simulink library "Garbage"  (R2023a) (continued)
== system Garbage/NOT_USED_Decoder_8_to_256 == (continued)
  xyz1/5 -> Lo`gical Operator6/1, Logical Operator127/5, Logical Operator1/5, Logical Operator2/5, Logical Operator3/5, Logical Operator4/5, Logical Operator5/5, Logical Operator6/5, Logical Operator7/5, Logical Operator256/5, Logical Operator20/5, Logical Operator16/5, Logical Operator24/5, Logical Operator25/5, Logical Operator26/5, Logical Operator27/5, Logical Operator28/5, Logical Operator22/5, Logical Operator23/5, Logical Operator36/5, Logical Operator32/5, Logical Operator40/5, Logical Operator41/5, Logical Operator42/5, Logical Operator43/5, Logical Operator37/5, Logical Operator38/5, Logical Operator39/5, Logical Operator52/5, Logical Operator48/5, Logical Operator56/5, Logical Operator57/5, Logical Operator58/5, Logical Operator53/5, Logical Operator54/5, Logical Operator55/5, Logical Operator68/5, Logical Operator64/5, Logical Operator253/5, Logical Operator76/5, Logical Operator89/5, Logical Operator67/5, Logical Operator69/5, Logical Operator70/5, Logical Operator71/5, Logical Operator77/5, Logical Operator72/5, Logical Operator81/5, Logical Operator84/5, Logical Operator74/5, Logical Operator75/5, Logical Operator78/5, Logical Operator79/5, Logical Operator80/5, Logical Operator96/5, Logical Operator92/5, Logical Operator101/5, Logical Operator93/5, Logical Operator94/5, Logical Operator95/5, Logical Operator97/5, Logical Operator98/5, Logical Operator99/5, Logical Operator115/5, Logical Operator109/5, Logical Operator113/5, Logical Operator249/5, Logical Operator114/5, Logical Operator116/5, Logical Operator117/5, Logical Operator118/5, Logical Operator136/5, Logical Operator195/5, Logical Operator133/5, Logical Operator134/5, Logical Operator135/5, Logical Operator137/5, Logical Operator138/5, Logical Operator139/5, Logical Operator145/5, Logical Operator156/5, Logical Operator157/5, Logical Operator141/5, Logical Operator142/5, Logical Operator143/5, Logical Operator146/5, Logical Operator147/5, Logical Operator148/5, Logical Operator172/5, Logical Operator173/5, Logical Operator174/5, Logical Operator159/5, Logical Operator160/5, Logical Operator161/5, Logical Operator163/5, Logical Operator164/5, Logical Operator190/5, Logical Operator245/5, Logical Operator191/5, Logical Operator192/5, Logical Operator176/5, Logical Operator178/5, Logical Operator179/5, Logical Operator181/5, Logical Operator252/5, Logical Operator257/5, Logical Operator244/5, Logical Operator258/5, Logical Operator259/5, Logical Operator197/5, Logical Operator198/5, Logical Operator199/5, Logical Operator216/5, Logical Operator217/5, Logical Operator218/5, Logical Operator220/5, Logical Operator221/5, Logical Operator205/5, Logical Operator243/5, Logical Operator206/5, Logical Operator233/5, Logical Operator234/5, Logical Operator235/5, Logical Operator236/5, Logical Operator237/5, Logical Operator238/5, Logical Operator242/5, Logical Operator223/5, Logical Operator110/5  "<signal5>"
  xyz1/7 -> Logical Operator82/1, Logical Operator127/7, Logical Operator1/7, Logical Operator4/7, Logical Operator5/7, Logical Operator8/7, Logical Operator9/7, Logical Operator12/7, Logical Operator13/7, Logical Operator256/7, Logical Operator20/7, Logical Operator25/7, Logical Operator26/7, Logical Operator29/7, Logical Operator30/7, Logical Operator18/7, Logical Operator19/7, Logical Operator22/7, Logical Operator23/7, Logical Operator40/7, Logical Operator41/7, Logical Operator44/7, Logical Operator45/7, Logical Operator33/7, Logical Operator34/7, Logical Operator37/7, Logical Operator38/7, Logical Operator48/7, Logical Operator56/7, Logical Operator59/7, Logical Operator60/7, Logical Operator63/7, Logical Operator49/7, Logical Operator53/7, Logical Operator54/7, Logical Operator64/7, Logical Operator253/7, Logical Operator100/7, Logical Operator111/7, Logical Operator130/7, Logical Operator131/7, Logical Operator67/7, Logical Operator69/7, Logical Operator77/7, Logical Operator72/7, Logical Operator85/7, Logical Operator86/7, Logical Operator251/7, Logical Operator90/7, Logical Operator74/7, Logical Operator75/7, Logical Operator80/7, Logical Operator96/7, Logical Operator102/7, Logical Operator103/7, Logical Operator250/7, Logical Operator106/7, Logical Operator93/7, Logical Operator94/7, Logical Operator98/7, Logical Operator99/7, Logical Operator119/7, Logical Operator120/7, Logical Operator124/7, Logical Operator125/7, Logical Operator113/7, Logical Operator116/7, Logical Operator117/7, Logical Operator132/7, Logical Operator144/7, Logical Operator177/7, Logical Operator188/7, Logical Operator195/7, Logical Operator133/7, Logical Operator137/7, Logical Operator138/7, Logical Operator248/7, Logical Operator140/7, Logical Operator151/7, Logical Operator152/7, Logical Operator156/7, Logical Operator157/7, Logical Operator143/7, Logical Operator146/7, Logical Operator162/7, Logical Operator158/7, Logical Operator169/7, Logical Operator170/7, Logical Operator172/7, Logical Operator173/7, Logical Operator160/7, Logical Operator161/7, Logical Operator165/7, Logical Operator180/7, Logical Operator185/7, Logical Operator186/7, Logical Operator190/7, Logical Operator245/7, Logical Operator176/7, Logical Operator178/7, Logical Operator182/7, Logical Operator183/7, Logical Operator208/7, Logical Operator219/7, Logical Operator252/7, Logical Operator257/7, Logical Operator259/7, Logical Operator197/7, Logical Operator201/7, Logical Operator202/7, Logical Operator204/7, Logical Operator213/7, Logical Operator216/7, Logical Operator217/7, Logical Operator221/7, Logical Operator205/7, Logical Operator207/7, Logical Operator210/7, Logical Operator226/7, Logical Operator222/7, Logical Operator233/7, Logical Operator234/7, Logical Operator237/7, Logical Operator238/7, Logical Operator224/7, Logical Operator225/7, Logical Operator240/7, Logical Operator239/7, Logical Operator110/7  "<signal7>"
  xyz1/6 -> Logical Operator112/1, Logical Operator127/6, Logical Operator1/6, Logical Operator2/6, Logical Operator3/6, Logical Operator8/6, Logical Operator9/6, Logical Operator10/6, Logical Operator11/6, Logical Operator256/6, Logical Operator20/6, Logical Operator16/6, Logical Operator24/6, Logical Operator29/6, Logical Operator30/6, Logical Operator31/6, Logical Operator17/6, Logical Operator22/6, Logical Operator23/6, Logical Operator36/6, Logical Operator32/6, Logical Operator44/6, Logical Operator45/6, Logical Operator46/6, Logical Operator47/6, Logical Operator37/6, Logical Operator38/6, Logical Operator39/6, Logical Operator52/6, Logical Operator59/6, Logical Operator60/6, Logical Operator61/6, Logical Operator62/6, Logical Operator53/6, Logical Operator54/6, Logical Operator55/6, Logical Operator68/6, Logical Operator100/6, Logical Operator111/6, Logical Operator123/6, Logical Operator129/6, Logical Operator67/6, Logical Operator69/6, Logical Operator70/6, Logical Operator71/6, Logical Operator85/6, Logical Operator86/6, Logical Operator87/6, Logical Operator88/6, Logical Operator74/6, Logical Operator75/6, Logical Operator78/6, Logical Operator79/6, Logical Operator102/6, Logical Operator103/6, Logical Operator104/6, Logical Operator105/6, Logical Operator93/6, Logical Operator94/6, Logical Operator95/6, Logical Operator97/6, Logical Operator119/6, Logical Operator120/6, Logical Operator121/6, Logical Operator122/6, Logical Operator113/6, Logical Operator249/6, Logical Operator114/6, Logical Operator132/6, Logical Operator144/6, Logical Operator155/6, Logical Operator166/6, Logical Operator195/6, Logical Operator133/6, Logical Operator134/6, Logical Operator135/6, Logical Operator248/6, Logical Operator140/6, Logical Operator149/6, Logical Operator150/6, Logical Operator156/6, Logical Operator157/6, Logical Operator141/6, Logical Operator142/6, Logical Operator162/6, Logical Operator158/6, Logical Operator167/6, Logical Operator168/6, Logical Operator172/6, Logical Operator173/6, Logical Operator174/6, Logical Operator159/6, Logical Operator165/6, Logical Operator180/6, Logical Operator175/6, Logical Operator184/6, Logical Operator190/6, Logical Operator245/6, Logical Operator191/6, Logical Operator192/6, Logical Operator182/6, Logical Operator183/6, Logical Operator200/6, Logical Operator196/6, Logical Operator252/6, Logical Operator257/6, Logical Operator244/6, Logical Operator258/6, Logical Operator201/6, Logical Operator202/6, Logical Operator203/6, Logical Operator209/6, Logical Operator216/6, Logical Operator217/6, Logical Operator218/6, Logical Operator220/6, Logical Operator207/6, Logical Operator210/6, Logical Operator211/6, Logical Operator212/6, Logical Operator233/6, Logical Operator234/6, Logical Operator235/6, Logical Operator236/6, Logical Operator224/6, Logical Operator225/6, Logical Operator227/6, Logical Operator228/6, Logical Operator110/6  "<signal6>"
  Lo`gical Operator6/1 -> Logical Operator8/5, Logical Operator9/5, Logical Operator10/5, Logical Operator11/5, Logical Operator12/5, Logical Operator13/5, Logical Operator14/5, Logical Operator15/5, Logical Operator29/5, Logical Operator30/5, Logical Operator31/5, Logical Operator17/5, Logical Operator18/5, Logical Operator19/5, Logical Operator21/5, Logical Operator255/5, Logical Operator44/5, Logical Operator45/5, Logical Operator46/5, Logical Operator47/5, Logical Operator33/5, Logical Operator34/5, Logical Operator35/5, Logical Operator254/5, Logical Operator59/5, Logical Operator60/5, Logical Operator61/5, Logical Operator62/5, Logical Operator63/5, Logical Operator49/5, Logical Operator50/5, Logical Operator51/5, Logical Operator100/5, Logical Operator111/5, Logical Operator123/5, Logical Operator129/5, Logical Operator130/5, Logical Operator131/5, Logical Operator65/5, Logical Operator66/5, Logical Operator85/5, Logical Operator86/5, Logical Operator87/5, Logical Operator88/5, Logical Operator251/5, Logical Operator90/5, Logical Operator91/5, Logical Operator73/5, Logical Operator102/5, Logical Operator103/5, Logical Operator104/5, Logical Operator105/5, Logical Operator250/5, Logical Operator106/5, Logical Operator107/5, Logical Operator108/5, Logical Operator119/5, Logical Operator120/5, Logical Operator121/5, Logical Operator122/5, Logical Operator124/5, Logical Operator125/5, Logical Operator126/5, Logical Operator128/5, Logical Operator132/5, Logical Operator144/5, Logical Operator155/5, Logical Operator166/5, Logical Operator177/5, Logical Operator188/5, Logical Operator193/5, Logical Operator194/5, Logical Operator248/5, Logical Operator140/5, Logical Operator149/5, Logical Operator150/5, Logical Operator151/5, Logical Operator152/5, Logical Operator153/5, Logical Operator154/5, Logical Operator162/5, Logical Operator158/5, Logical Operator167/5, Logical Operator168/5, Logical Operator169/5, Logical Operator170/5, Logical Operator246/5, Logical Operator171/5, Logical Operator165/5, Logical Operator180/5, Logical Operator175/5, Logical Operator184/5, Logical Operator185/5, Logical Operator186/5, Logical Operator187/5, Logical Operator189/5, Logical Operator182/5, Logical Operator183/5, Logical Operator200/5, Logical Operator196/5, Logical Operator208/5, Logical Operator219/5, Logical Operator230/5, Logical Operator241/5, Logical Operator201/5, Logical Operator202/5, Logical Operator203/5, Logical Operator209/5, Logical Operator204/5, Logical Operator213/5, Logical Operator214/5, Logical Operator215/5, Logical Operator207/5, Logical Operator210/5, Logical Operator211/5, Logical Operator212/5, Logical Operator226/5, Logical Operator222/5, Logical Operator231/5, Logical Operator232/5, Logical Operator224/5, Logical Operator225/5, Logical Operator227/5, Logical Operator228/5, Logical Operator240/5, Logical Operator239/5, Logical Operator229/5, Logical Operator247/5
  Logical Operator247/1 -> Select_000(1b)/1
  Logical Operator229/1 -> Select_001(1b)/1
  Logical Operator239/1 -> Select_002(1b)/1
  Logical Operator240/1 -> Select_003(1b)/1
  Logical Operator228/1 -> Select_004(1b)/1
  Logical Operator227/1 -> Select_005(1b)/1
  Logical Operator225/1 -> Select_006(1b)/1
  Logical Operator224/1 -> Select_007(1b)/1
  Logical Operator223/1 -> Select_008(1b)/1
  Logical Operator242/1 -> Select_009(1b)/1
  Logical Operator238/1 -> Select_010(1b)/1
  Logical Operator237/1 -> Select_011(1b)/1
  Logical Operator234/1 -> Select_014(1b)/1
  Logical Operator235/1 -> Select_013(1b)/1
  Logical Operator236/1 -> Select_012(1b)/1
  Logical Operator233/1 -> Select_015(1b)/1
  Lo`gical Operator3/1 -> Logical Operator256/4, Logical Operator20/4, Logical Operator16/4, Logical Operator24/4, Logical Operator25/4, Logical Operator26/4, Logical Operator27/4, Logical Operator28/4, Logical Operator29/4, Logical Operator30/4, Logical Operator31/4, Logical Operator17/4, Logical Operator18/4, Logical Operator19/4, Logical Operator21/4, Logical Operator255/4, Logical Operator37/4, Logical Operator38/4, Logical Operator39/4, Logical Operator52/4, Logical Operator48/4, Logical Operator56/4, Logical Operator57/4, Logical Operator58/4, Logical Operator59/4, Logical Operator60/4, Logical Operator61/4, Logical Operator62/4, Logical Operator63/4, Logical Operator49/4, Logical Operator50/4, Logical Operator51/4, Logical Operator67/4, Logical Operator69/4, Logical Operator70/4, Logical Operator71/4, Logical Operator77/4, Logical Operator72/4, Logical Operator81/4, Logical Operator84/4, Logical Operator85/4, Logical Operator86/4, Logical Operator87/4, Logical Operator88/4, Logical Operator251/4, Logical Operator90/4, Logical Operator91/4, Logical Operator73/4, Logical Operator93/4, Logical Operator94/4, Logical Operator95/4, Logical Operator97/4, Logical Operator98/4, Logical Operator99/4, Logical Operator115/4, Logical Operator109/4, Logical Operator119/4, Logical Operator120/4, Logical Operator121/4, Logical Operator122/4, Logical Operator124/4, Logical Operator125/4, Logical Operator126/4, Logical Operator128/4, Logical Operator195/4, Logical Operator133/4, Logical Operator134/4, Logical Operator135/4, Logical Operator137/4, Logical Operator138/4, Logical Operator139/4, Logical Operator145/4, Logical Operator248/4, Logical Operator140/4, Logical Operator149/4, Logical Operator150/4, Logical Operator151/4, Logical Operator152/4, Logical Operator153/4, Logical Operator154/4, Logical Operator172/4, Logical Operator173/4, Logical Operator174/4, Logical Operator159/4, Logical Operator160/4, Logical Operator161/4, Logical Operator163/4, Logical Operator164/4, Logical Operator165/4, Logical Operator180/4, Logical Operator175/4, Logical Operator184/4, Logical Operator185/4, Logical Operator186/4, Logical Operator187/4, Logical Operator189/4, Logical Operator252/4, Logical Operator257/4, Logical Operator244/4, Logical Operator258/4, Logical Operator259/4, Logical Operator197/4, Logical Operator198/4, Logical Operator199/4, Logical Operator201/4, Logical Operator202/4, Logical Operator203/4, Logical Operator209/4, Logical Operator204/4, Logical Operator213/4, Logical Operator214/4, Logical Operator215/4, Logical Operator233/4, Logical Operator234/4, Logical Operator235/4, Logical Operator236/4, Logical Operator237/4, Logical Operator238/4, Logical Operator242/4, Logical Operator223/4, Logical Operator224/4, Logical Operator225/4, Logical Operator227/4, Logical Operator228/4, Logical Operator240/4, Logical Operator239/4, Logical Operator229/4, Logical Operator247/4
  xyz1/4 -> Lo`gical Operator3/1, Logical Operator127/4, Logical Operator1/4, Logical Operator2/4, Logical Operator3/4, Logical Operator4/4, Logical Operator5/4, Logical Operator6/4, Logical Operator7/4, Logical Operator8/4, Logical Operator9/4, Logical Operator10/4, Logical Operator11/4, Logical Operator12/4, Logical Operator13/4, Logical Operator14/4, Logical Operator15/4, Logical Operator22/4, Logical Operator23/4, Logical Operator36/4, Logical Operator32/4, Logical Operator40/4, Logical Operator41/4, Logical Operator42/4, Logical Operator43/4, Logical Operator44/4, Logical Operator45/4, Logical Operator46/4, Logical Operator47/4, Logical Operator33/4, Logical Operator34/4, Logical Operator35/4, Logical Operator254/4, Logical Operator53/4, Logical Operator54/4, Logical Operator55/4, Logical Operator68/4, Logical Operator64/4, Logical Operator253/4, Logical Operator76/4, Logical Operator89/4, Logical Operator100/4, Logical Operator111/4, Logical Operator123/4, Logical Operator129/4, Logical Operator130/4, Logical Operator131/4, Logical Operator65/4, Logical Operator66/4, Logical Operator74/4, Logical Operator75/4, Logical Operator78/4, Logical Operator79/4, Logical Operator80/4, Logical Operator96/4, Logical Operator92/4, Logical Operator101/4, Logical Operator102/4, Logical Operator103/4, Logical Operator104/4, Logical Operator105/4, Logical Operator250/4, Logical Operator106/4, Logical Operator107/4, Logical Operator108/4, Logical Operator113/4, Logical Operator249/4, Logical Operator114/4, Logical Operator116/4, Logical Operator117/4, Logical Operator118/4, Logical Operator136/4, Logical Operator132/4, Logical Operator144/4, Logical Operator155/4, Logical Operator166/4, Logical Operator177/4, Logical Operator188/4, Logical Operator193/4, Logical Operator194/4, Logical Operator156/4, Logical Operator157/4, Logical Operator141/4, Logical Operator142/4, Logical Operator143/4, Logical Operator146/4, Logical Operator147/4, Logical Operator148/4, Logical Operator162/4, Logical Operator158/4, Logical Operator167/4, Logical Operator168/4, Logical Operator169/4, Logical Operator170/4, Logical Operator246/4, Logical Operator171/4, Logical Operator190/4, Logical Operator245/4, Logical Operator191/4, Logical Operator192/4, Logical Operator176/4, Logical Operator178/4, Logical Operator179/4, Logical Operator181/4, Logical Operator182/4, Logical Operator183/4, Logical Operator200/4, Logical Operator196/4, Logical Operator208/4, Logical Operator219/4, Logical Operator230/4, Logical Operator241/4, Logical Operator216/4, Logical Operator217/4, Logical Operator218/4, Logical Operator220/4, Logical Operator221/4, Logical Operator205/4, Logical Operator243/4, Logical Operator206/4, Logical Operator207/4, Logical Operator210/4, Logical Operator211/4, Logical Operator212/4, Logical Operator226/4, Logical Operator222/4, Logical Operator231/4, Logical Operator232/4, Logical Operator110/4  "<signal4>"
  Lo`gical Operator5/1 -> Logical Operator22/3, Logical Operator23/3, Logical Operator36/3, Logical Operator32/3, Logical Operator40/3, Logical Operator41/3, Logical Operator42/3, Logical Operator43/3, Logical Operator44/3, Logical Operator45/3, Logical Operator46/3, Logical Operator47/3, Logical Operator33/3, Logical Operator34/3, Logical Operator35/3, Logical Operator254/3, Logical Operator37/3, Logical Operator38/3, Logical Operator39/3, Logical Operator52/3, Logical Operator48/3, Logical Operator56/3, Logical Operator57/3, Logical Operator58/3, Logical Operator59/3, Logical Operator60/3, Logical Operator61/3, Logical Operator62/3, Logical Operator63/3, Logical Operator49/3, Logical Operator50/3, Logical Operator51/3, Logical Operator74/3, Logical Operator75/3, Logical Operator78/3, Logical Operator79/3, Logical Operator80/3, Logical Operator96/3, Logical Operator92/3, Logical Operator101/3, Logical Operator102/3, Logical Operator103/3, Logical Operator104/3, Logical Operator105/3, Logical Operator250/3, Logical Operator106/3, Logical Operator107/3, Logical Operator108/3, Logical Operator93/3, Logical Operator94/3, Logical Operator95/3, Logical Operator97/3, Logical Operator98/3, Logical Operator99/3, Logical Operator115/3, Logical Operator109/3, Logical Operator119/3, Logical Operator120/3, Logical Operator121/3, Logical Operator122/3, Logical Operator124/3, Logical Operator125/3, Logical Operator126/3, Logical Operator128/3, Logical Operator156/3, Logical Operator157/3, Logical Operator141/3, Logical Operator142/3, Logical Operator143/3, Logical Operator146/3, Logical Operator147/3, Logical Operator148/3, Logical Operator162/3, Logical Operator158/3, Logical Operator167/3, Logical Operator168/3, Logical Operator169/3, Logical Operator170/3, Logical Operator246/3, Logical Operator171/3, Logical Operator172/3, Logical Operator173/3, Logical Operator174/3, Logical Operator159/3, Logical Operator160/3, Logical Operator161/3, Logical Operator163/3, Logical Operator164/3, Logical Operator165/3, Logical Operator180/3, Logical Operator175/3, Logical Operator184/3, Logical Operator185/3, Logical Operator186/3, Logical Operator187/3, Logical Operator189/3, Logical Operator216/3, Logical Operator217/3, Logical Operator218/3, Logical Operator220/3, Logical Operator221/3, Logical Operator205/3, Logical Operator243/3, Logical Operator206/3, Logical Operator207/3, Logical Operator210/3, Logical Operator211/3, Logical Operator212/3, Logical Operator226/3, Logical Operator222/3, Logical Operator231/3, Logical Operator232/3, Logical Operator233/3, Logical Operator234/3, Logical Operator235/3, Logical Operator236/3, Logical Operator237/3, Logical Operator238/3, Logical Operator242/3, Logical Operator223/3, Logical Operator224/3, Logical Operator225/3, Logical Operator227/3, Logical Operator228/3, Logical Operator240/3, Logical Operator239/3, Logical Operator229/3, Logical Operator247/3
  Lo`gical Operator9/1 -> Logical Operator113/1, Logical Operator249/1, Logical Operator114/1, Logical Operator116/1, Logical Operator117/1, Logical Operator118/1, Logical Operator136/1, Logical Operator132/1, Logical Operator144/1, Logical Operator155/1, Logical Operator166/1, Logical Operator177/1, Logical Operator188/1, Logical Operator193/1, Logical Operator194/1, Logical Operator195/1, Logical Operator133/1, Logical Operator134/1, Logical Operator135/1, Logical Operator137/1, Logical Operator138/1, Logical Operator139/1, Logical Operator145/1, Logical Operator248/1, Logical Operator140/1, Logical Operator149/1, Logical Operator150/1, Logical Operator151/1, Logical Operator152/1, Logical Operator153/1, Logical Operator154/1, Logical Operator156/1, Logical Operator157/1, Logical Operator141/1, Logical Operator142/1, Logical Operator143/1, Logical Operator146/1, Logical Operator147/1, Logical Operator148/1, Logical Operator162/1, Logical Operator158/1, Logical Operator167/1, Logical Operator168/1, Logical Operator169/1, Logical Operator170/1, Logical Operator246/1, Logical Operator171/1, Logical Operator172/1, Logical Operator173/1, Logical Operator174/1, Logical Operator159/1, Logical Operator160/1, Logical Operator161/1, Logical Operator163/1, Logical Operator164/1, Logical Operator165/1, Logical Operator180/1, Logical Operator175/1, Logical Operator184/1, Logical Operator185/1, Logical Operator186/1, Logical Operator187/1, Logical Operator189/1, Logical Operator190/1, Logical Operator245/1, Logical Operator191/1, Logical Operator192/1, Logical Operator176/1, Logical Operator178/1, Logical Operator179/1, Logical Operator181/1, Logical Operator182/1, Logical Operator183/1, Logical Operator200/1, Logical Operator196/1, Logical Operator208/1, Logical Operator219/1, Logical Operator230/1, Logical Operator241/1, Logical Operator252/1, Logical Operator257/1, Logical Operator244/1, Logical Operator258/1, Logical Operator259/1, Logical Operator197/1, Logical Operator198/1, Logical Operator199/1, Logical Operator201/1, Logical Operator202/1, Logical Operator203/1, Logical Operator209/1, Logical Operator204/1, Logical Operator213/1, Logical Operator214/1, Logical Operator215/1, Logical Operator216/1, Logical Operator217/1, Logical Operator218/1, Logical Operator220/1, Logical Operator221/1, Logical Operator205/1, Logical Operator243/1, Logical Operator206/1, Logical Operator207/1, Logical Operator210/1, Logical Operator211/1, Logical Operator212/1, Logical Operator226/1, Logical Operator222/1, Logical Operator231/1, Logical Operator232/1, Logical Operator233/1, Logical Operator234/1, Logical Operator235/1, Logical Operator236/1, Logical Operator237/1, Logical Operator238/1, Logical Operator242/1, Logical Operator223/1, Logical Operator224/1, Logical Operator225/1, Logical Operator227/1, Logical Operator228/1, Logical Operator240/1, Logical Operator239/1, Logical Operator229/1, Logical Operator247/1, Logical Operator110/1
  xyz1/2 -> Lo`gical Operator1/1, Logical Operator127/2, Logical Operator1/2, Logical Operator2/2, Logical Operator3/2, Logical Operator4/2, Logical Operator5/2, Logical Operator6/2, Logical Operator7/2, Logical Operator8/2, Logical Operator9/2, Logical Operator10/2, Logical Operator11/2, Logical Operator12/2, Logical Operator13/2, Logical Operator14/2, Logical Operator15/2, Logical Operator256/2, Logical Operator20/2, Logical Operator16/2, Logical Operator24/2, Logical Operator25/2, Logical Operator26/2, Logical Operator27/2, Logical Operator28/2, Logical Operator29/2, Logical Operator30/2, Logical Operator31/2, Logical Operator17/2, Logical Operator18/2, Logical Operator19/2, Logical Operator21/2, Logical Operator255/2, Logical Operator22/2, Logical Operator23/2, Logical Operator36/2, Logical Operator32/2, Logical Operator40/2, Logical Operator41/2, Logical Operator42/2, Logical Operator43/2, Logical Operator44/2, Logical Operator45/2, Logical Operator46/2, Logical Operator47/2, Logical Operator33/2, Logical Operator34/2, Logical Operator35/2, Logical Operator254/2, Logical Operator37/2, Logical Operator38/2, Logical Operator39/2, Logical Operator52/2, Logical Operator48/2, Logical Operator56/2, Logical Operator57/2, Logical Operator58/2, Logical Operator59/2, Logical Operator60/2, Logical Operator61/2, Logical Operator62/2, Logical Operator63/2, Logical Operator49/2, Logical Operator50/2, Logical Operator51/2, Logical Operator113/2, Logical Operator249/2, Logical Operator114/2, Logical Operator116/2, Logical Operator117/2, Logical Operator118/2, Logical Operator136/2, Logical Operator132/2, Logical Operator144/2, Logical Operator155/2, Logical Operator166/2, Logical Operator177/2, Logical Operator188/2, Logical Operator193/2, Logical Operator194/2, Logical Operator195/2, Logical Operator133/2, Logical Operator134/2, Logical Operator135/2, Logical Operator137/2, Logical Operator138/2, Logical Operator139/2, Logical Operator145/2, Logical Operator248/2, Logical Operator140/2, Logical Operator149/2, Logical Operator150/2, Logical Operator151/2, Logical Operator152/2, Logical Operator153/2, Logical Operator154/2, Logical Operator156/2, Logical Operator157/2, Logical Operator141/2, Logical Operator142/2, Logical Operator143/2, Logical Operator146/2, Logical Operator147/2, Logical Operator148/2, Logical Operator162/2, Logical Operator158/2, Logical Operator167/2, Logical Operator168/2, Logical Operator169/2, Logical Operator170/2, Logical Operator246/2, Logical Operator171/2, Logical Operator172/2, Logical Operator173/2, Logical Operator174/2, Logical Operator159/2, Logical Operator160/2, Logical Operator161/2, Logical Operator163/2, Logical Operator164/2, Logical Operator165/2, Logical Operator180/2, Logical Operator175/2, Logical Operator184/2, Logical Operator185/2, Logical Operator186/2, Logical Operator189/2, Logical Operator187/2, Logical Operator110/2  "<signal2>"
  Logical Operator194/1 -> Select_112(1b)/1
  Logical Operator193/1 -> Select_113(1b)/1
  Logical Operator188/1 -> Select_114(1b)/1
  Logical Operator177/1 -> Select_115(1b)/1
  Logical Operator166/1 -> Select_116(1b)/1
  Logical Operator155/1 -> Select_117(1b)/1
  Logical Operator144/1 -> Select_118(1b)/1
  Logical Operator132/1 -> Select_119(1b)/1
  Logical Operator136/1 -> Select_120(1b)/1
  Logical Operator118/1 -> Select_121(1b)/1
  Logical Operator117/1 -> Select_122(1b)/1
  Logical Operator116/1 -> Select_123(1b)/1
  Logical Operator113/1 -> Select_126(1b)/1
  Logical Operator249/1 -> Select_125(1b)/1
  Logical Operator114/1 -> Select_124(1b)/1
  Logical Operator110/1 -> Select_127(1b)/1
  Logical Operator154/1 -> Select_096(1b)/1
  Logical Operator153/1 -> Select_097(1b)/1
  Logical Operator152/1 -> Select_098(1b)/1
  Logical Operator151/1 -> Select_099(1b)/1
  Logical Operator150/1 -> Select_100(1b)/1
  Logical Operator149/1 -> Select_101(1b)/1
  Logical Operator140/1 -> Select_102(1b)/1
  Logical Operator248/1 -> Select_103(1b)/1
  Logical Operator145/1 -> Select_104(1b)/1
  Logical Operator139/1 -> Select_105(1b)/1
  Logical Operator138/1 -> Select_106(1b)/1
  Logical Operator137/1 -> Select_107(1b)/1
  Logical Operator133/1 -> Select_110(1b)/1
  Logical Operator134/1 -> Select_109(1b)/1
  Logical Operator135/1 -> Select_108(1b)/1
  Logical Operator195/1 -> Select_111(1b)/1
  Logical Operator171/1 -> Select_080(1b)/1
  Logical Operator246/1 -> Select_081(1b)/1
  Logical Operator170/1 -> Select_082(1b)/1
  Logical Operator169/1 -> Select_083(1b)/1
  Logical Operator168/1 -> Select_084(1b)/1
  Logical Operator167/1 -> Select_085(1b)/1
  Logical Operator158/1 -> Select_086(1b)/1
  Logical Operator162/1 -> Select_087(1b)/1
  Logical Operator148/1 -> Select_088(1b)/1
  Logical Operator147/1 -> Select_089(1b)/1
  Logical Operator146/1 -> Select_090(1b)/1
  Logical Operator143/1 -> Select_091(1b)/1
  Logical Operator157/1 -> Select_094(1b)/1
  Logical Operator141/1 -> Select_093(1b)/1
  Logical Operator142/1 -> Select_092(1b)/1
  Logical Operator156/1 -> Select_095(1b)/1
  Logical Operator189/1 -> Select_064(1b)/1
  Logical Operator187/1 -> Select_065(1b)/1
  Logical Operator186/1 -> Select_066(1b)/1
  Logical Operator185/1 -> Select_067(1b)/1
  Logical Operator184/1 -> Select_068(1b)/1
  Logical Operator175/1 -> Select_069(1b)/1
  Logical Operator180/1 -> Select_070(1b)/1
  Logical Operator165/1 -> Select_071(1b)/1
  Logical Operator164/1 -> Select_072(1b)/1
  Logical Operator163/1 -> Select_073(1b)/1
  Logical Operator161/1 -> Select_074(1b)/1
  Logical Operator160/1 -> Select_075(1b)/1
  Logical Operator173/1 -> Select_078(1b)/1
  Logical Operator174/1 -> Select_077(1b)/1
  Logical Operator159/1 -> Select_076(1b)/1
  Logical Operator172/1 -> Select_079(1b)/1
  Lo`gical Operator1/1 -> Logical Operator53/2, Logical Operator54/2, Logical Operator55/2, Logical Operator68/2, Logical Operator64/2, Logical Operator253/2, Logical Operator76/2, Logical Operator89/2, Logical Operator100/2, Logical Operator111/2, Logical Operator123/2, Logical Operator129/2, Logical Operator130/2, Logical Operator131/2, Logical Operator65/2, Logical Operator66/2, Logical Operator67/2, Logical Operator69/2, Logical Operator70/2, Logical Operator71/2, Logical Operator77/2, Logical Operator72/2, Logical Operator81/2, Logical Operator84/2, Logical Operator85/2, Logical Operator86/2, Logical Operator87/2, Logical Operator88/2, Logical Operator251/2, Logical Operator90/2, Logical Operator91/2, Logical Operator73/2, Logical Operator74/2, Logical Operator75/2, Logical Operator78/2, Logical Operator79/2, Logical Operator80/2, Logical Operator96/2, Logical Operator92/2, Logical Operator101/2, Logical Operator102/2, Logical Operator103/2, Logical Operator104/2, Logical Operator105/2, Logical Operator250/2, Logical Operator106/2, Logical Operator107/2, Logical Operator108/2, Logical Operator93/2, Logical Operator94/2, Logical Operator95/2, Logical Operator97/2, Logical Operator98/2, Logical Operator99/2, Logical Operator115/2, Logical Operator109/2, Logical Operator119/2, Logical Operator120/2, Logical Operator121/2, Logical Operator122/2, Logical Operator124/2, Logical Operator125/2, Logical Operator126/2, Logical Operator128/2, Logical Operator190/2, Logical Operator245/2, Logical Operator191/2, Logical Operator192/2, Logical Operator176/2, Logical Operator178/2, Logical Operator179/2, Logical Operator181/2, Logical Operator182/2, Logical Operator183/2, Logical Operator200/2, Logical Operator196/2, Logical Operator208/2, Logical Operator219/2, Logical Operator230/2, Logical Operator241/2, Logical Operator252/2, Logical Operator257/2, Logical Operator244/2, Logical Operator258/2, Logical Operator259/2, Logical Operator197/2, Logical Operator198/2, Logical Operator199/2, Logical Operator201/2, Logical Operator202/2, Logical Operator203/2, Logical Operator209/2, Logical Operator204/2, Logical Operator213/2, Logical Operator214/2, Logical Operator215/2, Logical Operator216/2, Logical Operator217/2, Logical Operator218/2, Logical Operator220/2, Logical Operator221/2, Logical Operator205/2, Logical Operator243/2, Logical Operator206/2, Logical Operator207/2, Logical Operator210/2, Logical Operator211/2, Logical Operator212/2, Logical Operator226/2, Logical Operator222/2, Logical Operator231/2, Logical Operator232/2, Logical Operator233/2, Logical Operator234/2, Logical Operator235/2, Logical Operator236/2, Logical Operator237/2, Logical Operator238/2, Logical Operator242/2, Logical Operator223/2, Logical Operator224/2, Logical Operator225/2, Logical Operator227/2, Logical Operator228/2, Logical Operator240/2, Logical Operator239/2, Logical Operator229/2, Logical Operator247/2
  Addr(1B)/1 -> xyz1/1
